simulink model "FluxModel2015a_verify_Q_zhixin"  (Simulink 10.2)
settings: Solver=VariableStepAuto  StartTime=0.0  StopTime=0.5  FixedStep=Ts  RelTol=1e-3  EnableMultiTasking=on
values from neighbouring files (symbols this model uses):
  AngleEncoderEn = 1    [parameters.m]
  AngleSpdClosedLoopEnable = 1.0    (= 1)    [parameters.m]
  CurrentLoopMax = Q14*1.2    (= 19660.8)    [parameters.m]
  EstOutMax_Speed_Q = Q14*1.2    (= 19660.8)    [parameters.m]
  Factor1 = Q15*Ts*Rb/Lq_realvalue    [parameters.m]
  Factor2 = Q15*Ts*Rs_realvalue/Lq_realvalue    (= 655.34)    [parameters.m]
  fieldweaken_Max = 0    [parameters.m]
  fieldweaken_Min = -0.5*Q14    (= -8192)    [parameters.m]
  FieldWeakenEn = 0    [parameters.m]
  fluxF = Lam_realvalue/fluxB    [parameters.m]
  fluxF_Q14 = Q14*fluxF    [parameters.m]
  Friction = 0.003    [parameters.m]
  Ib = 1.5*In    (= 24)    [parameters.m]
  Id_Ki = Q14*CurrentLoopBandwidth*Rs    [parameters.m]
  Id_Kp = Q14*CurrentLoopBandwidth*Ld    [parameters.m]
  IFCurrDecreased = 1    [parameters.m]
  IFCurrStart = 8    [parameters.m]
  IFCurrTarget = 5    [parameters.m]
  IFIdref = 0    [parameters.m]
  initial_degree = 0/Np    (= 0)    [parameters.m]
  Iq_Ki = Q14*CurrentLoopBandwidth*Rs    [parameters.m]
  Iq_Kp = Q14*CurrentLoopBandwidth*Lq    [parameters.m]
  J_realvalue = 0.69e-4    (= 6.9e-05)    [parameters.m]
  KI_fieldweaken = 0.1*Q14    (= 1638.4)    [parameters.m]
  KP_fieldweaken = 1.0*Q14    (= 16384)    [parameters.m]
  LamObserverGain = 0.8    [parameters.m]
  Ld = Ld_realvalue/Lb    [parameters.m]
  Ld_Q14 = Q14*Ld_realvalue/Lb    [parameters.m]
  Ld_realvalue = 0.25e-3    (= 0.00025)    [parameters.m]
  LowPassFilterBandWidth_Emf_Q = Q14*LowPassFilterBandWidth_Emf    (= 4915.2)    [parameters.m]
  LowPassFilterCoeff_Emf = Q15*(1/(1+LowPassFilterBandWidth_Emf*TsPu))    (= 30944.2341498)    [parameters.m]
  LowPassFilterCoeff_Spd = Q15*(1/(1+LowPassFilterBandWidth_Speed*SpeedloopTs/tb))    (= 20123.2126159)    [parameters.m]
  Lq = Lq_realvalue/Lb    [parameters.m]
  Lq_realvalue = 0.25e-3    (= 0.00025)    [parameters.m]
  MOVE = 1    [parameters.m]
  Np = 5    [parameters.m]
  Omega0 = 0.4    [parameters.m]
  OverModulationEn = 0    [parameters.m]
  PLL_KI = Q14*PLL_Bandwidth*PLL_Bandwidth    (= 655.36)    [parameters.m]
  PLL_KP = Q14*2*PLL_Bandwidth    (= 6553.6)    [parameters.m]
  PLL_OUTMax_W = Q14*1.2    (= 19660.8)    [parameters.m]
  PWM_Period = 1000    [parameters.m]
  Q14 = 16384    [parameters.m]
  Q15 = 32767    [parameters.m]
  Rs = Rs_realvalue/Rb    [parameters.m]
  Rs_Q14 = Q14*Rs_realvalue/Rb    [parameters.m]
  Rs_realvalue = 0.08    [parameters.m]
  SMO_Gain = Q14*0.85    (= 13926.4)    [parameters.m]
  SMO_I_ERROR = Q14*0.05    (= 819.2)    [parameters.m]
  SpdClosedLoopEnable = 1.0    (= 1)    [parameters.m]
  Speed_Ki = Q14*SpeedLoopBandwidth    (= 327.68)    [parameters.m]
  Speed_Kp = Q14*SpeedLoopBandwidth*JPu/fluxF    [parameters.m]
  SpeedIncreased = 0.1*Wb    (= 314.159265359)    [parameters.m]
  SpeedloopTs = 1/SpeedloopFrequency    (= 0.001)    [parameters.m]
  SpeedOutmax = 1.2*Q14    (= 19660.8)    [parameters.m]
  SpeedSwitch = 0.6*Wb    (= 1884.95559215)    [parameters.m]
  SpeedTarget = 0.8*Wb    (= 2513.27412287)    [parameters.m]
  STOP = 0    [parameters.m]
  SVCM_GAIN_Q = Q15*SVCM_GAIN    (= 26213.6)    [parameters.m]
  tb = 1/Wb    (= 0.000318309886184)    [parameters.m]
  Tf = 0    [parameters.m]
  thres = 0.1    [parameters.m]
  Ts = 1/SwitchFrequency    (= 6.25e-05)    [parameters.m]
  TsPu = Ts/tb    (= 0.196349540849)    [parameters.m]
  Vb = Un/sqrt(3)    [parameters.m]
  Vdc = 36    [parameters.m]
  Wb = 2*pi*fn    (= 3141.59265359)    [parameters.m]
undefined symbols (not defined in the file or in its folder): DiscIntNeverNeededParam, EstOutMax_Theta, magnitude, sigbuilder_block
libraries: simulink x7, powerlib x5
(part 2 of 2)

== system FluxModel2015a_verify_Q_zhixin/CurrLoop&Observer/Subsystem ==
  err : Inport
  Constant14 : Constant  Value=2*pi
  Constant15 : Constant  Value=pi
  Divide4 : Product  Inputs=*/
  Gain5 : Gain  Gain=180
  Math Function6 : Math  Operator=rem  SaturateOnIntegerOverflow=on
  err_Theta : Outport
  err_Rad : Outport  Port=2
  wires:
  Divide4/1 -> Gain5/1
  Constant15/1 -> Divide4/2
  err/1 -> Math Function6/1
  Math Function6/1 -> err_Rad/1, Divide4/1  "err_Theta"
  Constant14/1 -> Math Function6/2
  Gain5/1 -> err_Theta/1

== system FluxModel2015a_verify_Q_zhixin/CurrLoop&Observer/Subsystem1 ==
  err : Inport
  Constant14 : Constant  Value=2*pi
  Constant15 : Constant  Value=pi
  Divide4 : Product  Inputs=*/
  Gain5 : Gain  Gain=180
  Math Function6 : Math  Operator=rem  SaturateOnIntegerOverflow=on
  err_Theta : Outport
  err_Rad : Outport  Port=2
  wires:
  Gain5/1 -> err_Theta/1
  Constant14/1 -> Math Function6/2
  Math Function6/1 -> Divide4/1, err_Rad/1  "err_Theta"
  err/1 -> Math Function6/1
  Constant15/1 -> Divide4/2
  Divide4/1 -> Gain5/1

== system FluxModel2015a_verify_Q_zhixin/CurrLoop&Observer/Subsystem2 ==
  err : Inport
  Constant14 : Constant  Value=2*pi
  Constant15 : Constant  Value=pi
  Divide4 : Product  Inputs=*/
  Gain5 : Gain  Gain=180
  Math Function6 : Math  Operator=rem  SaturateOnIntegerOverflow=on
  err_Theta : Outport
  err_Rad : Outport  Port=2
  wires:
  Divide4/1 -> Gain5/1
  Constant15/1 -> Divide4/2
  err/1 -> Math Function6/1
  Math Function6/1 -> err_Rad/1, Divide4/1  "err_Theta"
  Constant14/1 -> Math Function6/2
  Gain5/1 -> err_Theta/1

== system FluxModel2015a_verify_Q_zhixin/CurrLoop&Observer/Subsystem3 ==
  err : Inport
  Constant14 : Constant  Value=2*pi
  Constant15 : Constant  Value=pi
  Divide4 : Product  Inputs=*/
  Gain5 : Gain  Gain=180
  Math Function6 : Math  Operator=rem  SaturateOnIntegerOverflow=on
  err_Theta : Outport
  err_Rad : Outport  Port=2
  wires:
  Gain5/1 -> err_Theta/1
  Constant14/1 -> Math Function6/2
  Math Function6/1 -> Divide4/1, err_Rad/1  "err_Theta"
  err/1 -> Math Function6/1
  Constant15/1 -> Divide4/2
  Divide4/1 -> Gain5/1

== system FluxModel2015a_verify_Q_zhixin/CurrLoop&Observer/Subsystem4 ==
  err : Inport
  Constant14 : Constant  Value=2*pi
  Constant15 : Constant  Value=pi
  Divide4 : Product  Inputs=*/
  Gain5 : Gain  Gain=180
  Math Function6 : Math  Operator=rem  SaturateOnIntegerOverflow=on
  err_Theta : Outport
  err_Rad : Outport  Port=2
  wires:
  Divide4/1 -> Gain5/1
  Constant15/1 -> Divide4/2
  err/1 -> Math Function6/1
  Math Function6/1 -> err_Rad/1, Divide4/1  "err_Theta"
  Constant14/1 -> Math Function6/2
  Gain5/1 -> err_Theta/1

== system FluxModel2015a_verify_Q_zhixin/CurrLoop&Observer/Subsystem5 ==
  err : Inport
  Gain1 : Gain  Gain=Wb
  Gain2 : Gain  Gain=1/(2*pi)
  Gain3 : Gain  Gain=60/Np
  err_Spd : Outport
  err_Fhz : Outport  Port=2
  wires:
  err/1 -> Gain1/1
  Gain2/1 -> err_Fhz/1, Gain3/1
  Gain1/1 -> Gain2/1
  Gain3/1 -> err_Spd/1

== system FluxModel2015a_verify_Q_zhixin/CurrLoop&Observer/Subsystem6 ==
  err : Inport
  Gain1 : Gain  Gain=Wb
  Gain2 : Gain  Gain=1/(2*pi)
  Gain3 : Gain  Gain=60/Np
  err_Spd : Outport
  err_Fhz : Outport  Port=2
  wires:
  Gain3/1 -> err_Spd/1
  Gain1/1 -> Gain2/1
  Gain2/1 -> Gain3/1, err_Fhz/1
  err/1 -> Gain1/1

== system FluxModel2015a_verify_Q_zhixin/CurrLoop&Observer/Subsystem7 ==
  err : Inport
  Gain1 : Gain  Gain=Wb
  Gain2 : Gain  Gain=1/(2*pi)
  Gain3 : Gain  Gain=60/Np
  err_Spd : Outport
  err_Fhz : Outport  Port=2
  wires:
  err/1 -> Gain1/1
  Gain2/1 -> err_Fhz/1, Gain3/1
  Gain1/1 -> Gain2/1
  Gain3/1 -> err_Spd/1

== system FluxModel2015a_verify_Q_zhixin/CurrLoop&Observer/Subsystem8 ==
  err : Inport
  Gain1 : Gain  Gain=Wb
  Gain2 : Gain  Gain=1/(2*pi)
  Gain3 : Gain  Gain=60/Np
  err_Spd : Outport
  err_Fhz : Outport  Port=2
  wires:
  Gain3/1 -> err_Spd/1
  Gain1/1 -> Gain2/1
  Gain2/1 -> Gain3/1, err_Fhz/1
  err/1 -> Gain1/1

== system FluxModel2015a_verify_Q_zhixin/CurrLoop&Observer/Subsystem9 ==
  err : Inport
  Gain1 : Gain  Gain=Wb
  Gain2 : Gain  Gain=1/(2*pi)
  Gain3 : Gain  Gain=60/Np
  err_Spd : Outport
  err_Fhz : Outport  Port=2
  wires:
  err/1 -> Gain1/1
  Gain2/1 -> err_Fhz/1, Gain3/1
  Gain1/1 -> Gain2/1
  Gain3/1 -> err_Spd/1

== system FluxModel2015a_verify_Q_zhixin/CurrLoop&Observer/ai-park ==
  UD : Inport
  UQ : Inport  Port=2
  The : Inport  Port=3
  Add : Sum
  Cos : Trigonometry  Operator=cos
  Product{,1..3} (4 alike) : Product
  Sin : Trigonometry
  Subtract : Sum  Inputs=+-
  Valpha : Outport
  Vbeta : Outport  Port=2
  wires:
  UQ/1 -> Product3/1, Product1/1
  Product1/1 -> Subtract/2
  Product3/1 -> Add/2
  Cos/1 -> Product3/2, Product/2
  Sin/1 -> Product2/2, Product1/2
  Product/1 -> Subtract/1
  Product2/1 -> Add/1
  The/1 -> Sin/1, Cos/1
  UD/1 -> Product/1, Product2/1
  Subtract/1 -> Valpha/1
  Add/1 -> Vbeta/1

== system FluxModel2015a_verify_Q_zhixin/CurrLoop&Observer/clark ==
  Iabc : Inport
  Add : Sum  Inputs=+-
  Demux2 : Demux  Outputs=3
  Gain : Gain  Gain=18919
  Gain1 : Gain  Gain=1/Q15
  Ialpha : Outport
  Ibeta : Outport  Port=2
  wires:
  Demux2/3 -> Add/2
  Add/1 -> Gain/1
  Demux2/1 -> Ialpha/1
  Demux2/2 -> Add/1
  Gain/1 -> Gain1/1
  Iabc/1 -> Demux2/1
  Gain1/1 -> Ibeta/1

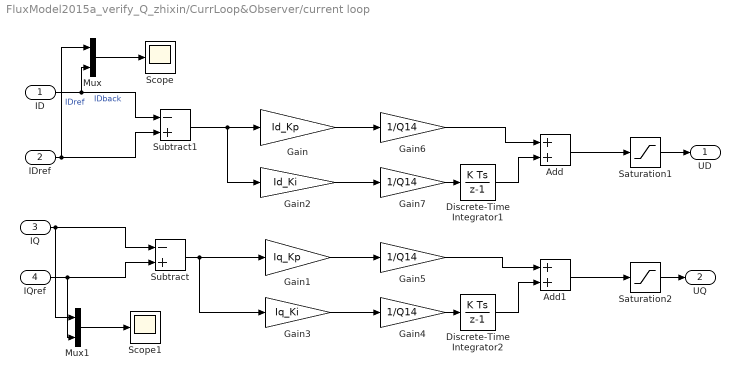
== system FluxModel2015a_verify_Q_zhixin/CurrLoop&Observer/current loop ==
  ID : Inport
  IDref : Inport  Port=2
  IQ : Inport  Port=3
  IQref : Inport  Port=4
  Add : Sum
  Add1 : Sum
  Discrete-Time Integrator1 : DiscreteIntegrator  gainval=1/tb  SampleTime=-1  LimitOutput=on  UpperSaturationLimit=CurrentLoopMax  LowerSaturationLimit=-CurrentLoopMax  ICPrevOutput=DiscIntNeverNeededParam  ICPrevScaledInput=DiscIntNeverNeededParam
  Discrete-Time Integrator2 : DiscreteIntegrator  gainval=1/tb  SampleTime=-1  LimitOutput=on  UpperSaturationLimit=CurrentLoopMax  LowerSaturationLimit=-CurrentLoopMax  ICPrevOutput=DiscIntNeverNeededParam  ICPrevScaledInput=DiscIntNeverNeededParam
  Gain : Gain  Gain=Id_Kp
  Gain1 : Gain  Gain=Iq_Kp
  Gain2 : Gain  Gain=Id_Ki
  Gain3 : Gain  Gain=Iq_Ki
  Gain{4..7} (4 alike) : Gain  Gain=1/Q14
  Mux : Mux  Inputs=2
  Mux1 : Mux  Inputs=2
  Saturation1 : Saturate  UpperLimit=CurrentLoopMax  LowerLimit=-CurrentLoopMax
  Saturation2 : Saturate  UpperLimit=CurrentLoopMax  LowerLimit=-CurrentLoopMax
  Scope : Scope
  Scope1 : Scope
  Subtract : Sum  Inputs=-+
  Subtract1 : Sum  Inputs=-+
  UD : Outport
  UQ : Outport  Port=2
  wires:
  Saturation1/1 -> UD/1
  Subtract1/1 -> Gain/1, Gain2/1
  Saturation2/1 -> UQ/1
  Gain1/1 -> Gain5/1
  Subtract/1 -> Gain3/1, Gain1/1
  Gain/1 -> Gain6/1
  Discrete-Time Integrator1/1 -> Add/2
  Discrete-Time Integrator2/1 -> Add1/2
  IQref/1 -> Mux1/2, Subtract/2
  IDref/1 -> Mux/1, Subtract1/2  "IDref"
  ID/1 -> Mux/2, Subtract1/1  "IDback"
  Mux/1 -> Scope/1
  IQ/1 -> Mux1/1, Subtract/1
  Mux1/1 -> Scope1/1
  Gain3/1 -> Gain4/1
  Add/1 -> Saturation1/1
  Add1/1 -> Saturation2/1
  Gain5/1 -> Add1/1
  Gain4/1 -> Discrete-Time Integrator2/1
  Gain6/1 -> Add/1
  Gain2/1 -> Gain7/1
  Gain7/1 -> Discrete-Time Integrator1/1

== system FluxModel2015a_verify_Q_zhixin/SVPWM/Subsystem ==
  Valpha : Inport
  Vbeta : Inport  Port=2
  Add : Sum  Inputs=+-
  Add1 : Sum  Inputs=+-
  Add2 : Sum  Inputs=+++
  Constant : Constant
  Constant1 : Constant  Value=0
  Gain : Gain  Gain=sqrt(3)
  Gain1 : Gain  Gain=-1
  Gain2 : Gain  Gain=2
  Gain3 : Gain  Gain=4
  Saturation : Saturate  UpperLimit=6  LowerLimit=1
  Switch : Switch  Criteria=u2 > Threshold
  Switch1 : Switch  Criteria=u2 > Threshold
  Switch2 : Switch  Criteria=u2 > Threshold
  N : Outport
  wires:
  Add2/1 -> Saturation/1
  Valpha/1 -> Gain/1
  Gain3/1 -> Add2/2
  Gain2/1 -> Add2/1
  Add1/1 -> Switch1/2
  Gain1/1 -> Add1/1
  Switch1/1 -> Gain3/1
  Switch2/1 -> Add2/3
  Switch/1 -> Gain2/1
  Add/1 -> Switch/2
  Gain/1 -> Gain1/1, Add/1
  Constant1/1 -> Switch/3, Switch1/3, Switch2/3
  Constant/1 -> Switch1/1, Switch2/1, Switch/1
  Vbeta/1 -> Add1/2, Add/2, Switch2/2
  Saturation/1 -> N/1

== system FluxModel2015a_verify_Q_zhixin/SVPWM/Subsystem1 ==
  T : Inport
  In2 : Inport  Port=2
  In3 : Inport  Port=3
  Vdc : Inport  Port=4
  Add : Sum
  Add1 : Sum  Inputs=+-
  Divide : Product  Inputs=**/
  Divide1 : Product  Inputs=**/
  Divide2 : Product  Inputs=**/
  Gain : Gain  Gain=1.732
  Gain1 : Gain  Gain=1.5
  Gain2 : Gain  Gain=0.5
  x : Outport
  Y : Outport  Port=2
  Z : Outport  Port=3
  wires:
  Divide2/1 -> Z/1
  Divide1/1 -> Y/1
  Divide/1 -> x/1
  In3/1 -> Gain1/1
  In2/1 -> Gain/1
  Add1/1 -> Divide2/2
  Add/1 -> Divide1/2
  Gain1/1 -> Add1/2, Add/2
  Gain2/1 -> Add1/1, Add/1
  Gain/1 -> Gain2/1, Divide/2
  Vdc/1 -> Divide/3, Divide1/3, Divide2/3
  T/1 -> Divide2/1, Divide1/1, Divide/1

== system FluxModel2015a_verify_Q_zhixin/SVPWM/Subsystem2 ==
  In1 : Inport
  In2 : Inport  Port=2
  In3 : Inport  Port=3
  In4 : Inport  Port=4
  Gain : Gain  Gain=-1
  Gain1 : Gain  Gain=-1
  Gain2 : Gain  Gain=-1
  Multiport Switch : MultiPortSwitch  Inputs=6
  Multiport Switch1 : MultiPortSwitch  Inputs=6
  T1 : Outport
  T2 : Outport  Port=2
  wires:
  Multiport Switch1/1 -> T2/1
  Multiport Switch/1 -> T1/1
  Gain2/1 -> Multiport Switch/7, Multiport Switch1/6
  Gain1/1 -> Multiport Switch1/3, Multiport Switch/5
  In2/1 -> Gain1/1, Multiport Switch/6, Multiport Switch1/4
  Gain/1 -> Multiport Switch1/7, Multiport Switch/4
  In3/1 -> Gain2/1, Multiport Switch1/2, Multiport Switch/3
  In4/1 -> Gain/1, Multiport Switch/2, Multiport Switch1/5
  In1/1 -> Multiport Switch/1, Multiport Switch1/1

== system FluxModel2015a_verify_Q_zhixin/SVPWM/Subsystem3 ==
  T1 : Inport
  T : Inport  Port=2
  T2 : Inport  Port=3
  Add : Sum  Inputs=--+
  Add1 : Sum
  Add2 : Sum
  Gain : Gain  Gain=0.25
  Gain1 : Gain  Gain=0.5
  Gain2 : Gain  Gain=0.5
  Saturation : Saturate  UpperLimit=Ts/2  LowerLimit=0
  Ta : Outport
  Tb : Outport  Port=2
  Tc : Outport  Port=3
  wires:
  T/1 -> Add/3
  Gain2/1 -> Add2/2
  Gain1/1 -> Add1/2
  Add/1 -> Gain/1
  T2/1 -> Add/2, Gain2/1
  T1/1 -> Add/1, Gain1/1
  Gain/1 -> Ta/1, Add1/1
  Add1/1 -> Tb/1, Add2/1
  Add2/1 -> Tc/1

== system FluxModel2015a_verify_Q_zhixin/SVPWM/Subsystem4 ==
  In1 : Inport
  In2 : Inport  Port=2
  In3 : Inport  Port=3
  In4 : Inport  Port=4
  Multiport Switch : MultiPortSwitch  Inputs=6
  Multiport Switch1 : MultiPortSwitch  Inputs=6
  Multiport Switch2 : MultiPortSwitch  Inputs=6
  Tcm1 : Outport
  Tcm2 : Outport  Port=2
  Tcm3 : Outport  Port=3
  wires:
  Multiport Switch2/1 -> Tcm3/1
  Multiport Switch1/1 -> Tcm2/1
  Multiport Switch/1 -> Tcm1/1
  In1/1 -> Multiport Switch2/1, Multiport Switch1/1, Multiport Switch/1
  In4/1 -> Multiport Switch1/3, Multiport Switch1/7, Multiport Switch2/2, Multiport Switch2/4, Multiport Switch/5, Multiport Switch/6
  In2/1 -> Multiport Switch/4, Multiport Switch/3, Multiport Switch1/2, Multiport Switch1/6, Multiport Switch2/5, Multiport Switch2/7
  In3/1 -> Multiport Switch/2, Multiport Switch/7, Multiport Switch1/4, Multiport Switch1/5, Multiport Switch2/3, Multiport Switch2/6

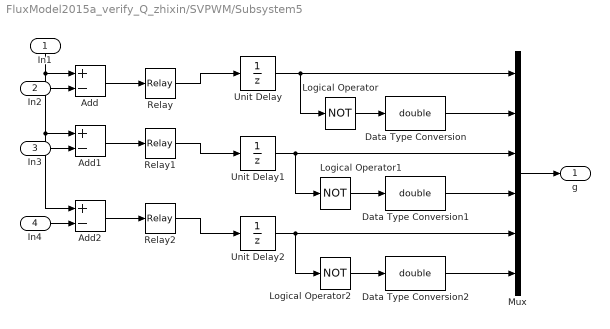
== system FluxModel2015a_verify_Q_zhixin/SVPWM/Subsystem5 ==
  In1 : Inport
  In2 : Inport  Port=2
  In3 : Inport  Port=3
  In4 : Inport  Port=4
  Add : Sum  Inputs=+-
  Add1 : Sum  Inputs=+-
  Add2 : Sum  Inputs=+-
  Data Type Conversion : DataTypeConversion  OutDataTypeStr=double
  Data Type Conversion1 : DataTypeConversion  OutDataTypeStr=double
  Data Type Conversion2 : DataTypeConversion  OutDataTypeStr=double
  Logical Operator : Logic  Operator=NOT  AllPortsSameDT=off  OutDataTypeStr=boolean
  Logical Operator1 : Logic  Operator=NOT  AllPortsSameDT=off  OutDataTypeStr=boolean
  Logical Operator2 : Logic  Operator=NOT  AllPortsSameDT=off  OutDataTypeStr=boolean
  Mux : Mux  Inputs=6
  Relay : Relay  OffSwitchValue=-eps
  Relay1 : Relay  OffSwitchValue=-eps
  Relay2 : Relay  OffSwitchValue=-eps
  Unit Delay : UnitDelay  SampleTime=-1  HasFrameUpgradeWarning=on
  Unit Delay1 : UnitDelay  SampleTime=-1  HasFrameUpgradeWarning=on
  Unit Delay2 : UnitDelay  SampleTime=-1  HasFrameUpgradeWarning=on
  g : Outport
  wires:
  In4/1 -> Add2/2
  In3/1 -> Add1/2
  In2/1 -> Add/2
  Relay2/1 -> Unit Delay2/1
  Relay1/1 -> Unit Delay1/1
  Relay/1 -> Unit Delay/1
  Unit Delay2/1 -> Mux/5, Logical Operator2/1
  Unit Delay1/1 -> Mux/3, Logical Operator1/1
  Unit Delay/1 -> Logical Operator/1, Mux/1
  Mux/1 -> g/1
  Data Type Conversion/1 -> Mux/2
  Data Type Conversion1/1 -> Mux/4
  Data Type Conversion2/1 -> Mux/6
  Logical Operator2/1 -> Data Type Conversion2/1
  Logical Operator1/1 -> Data Type Conversion1/1
  Logical Operator/1 -> Data Type Conversion/1
  Add1/1 -> Relay1/1
  Add2/1 -> Relay2/1
  Add/1 -> Relay/1
  In1/1 -> Add/1, Add2/1, Add1/1

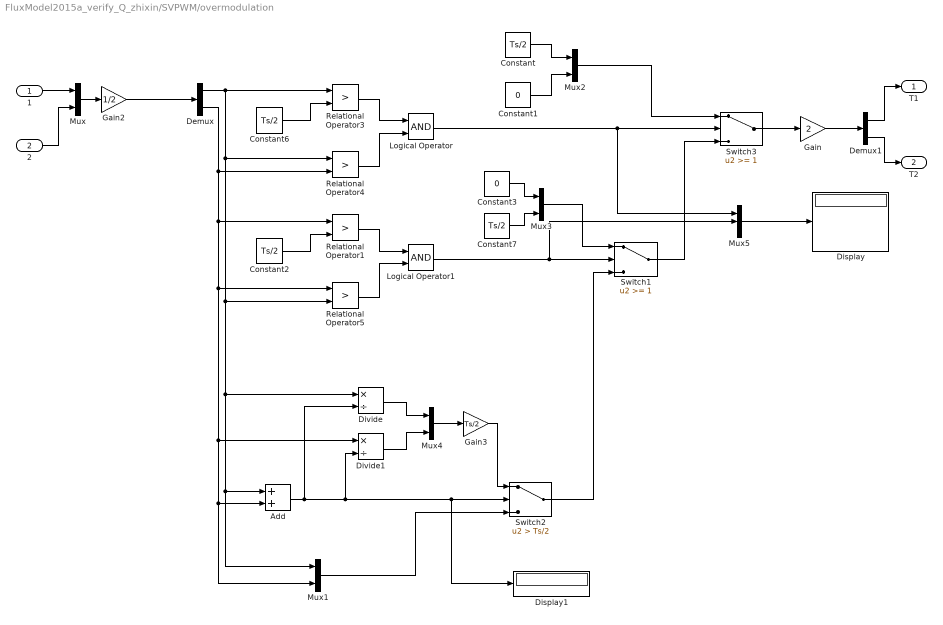
== system FluxModel2015a_verify_Q_zhixin/SVPWM/overmodulation ==
  1 : Inport
  2 : Inport  Port=2
  Add : Sum
  Constant{,2,6..7} (4 alike) : Constant  Value=Ts/2
  Constant1 : Constant  Value=0
  Constant3 : Constant  Value=0
  Demux : Demux  Outputs=2
  Demux1 : Demux  Outputs=2
  Display : Display
  Display1 : Display
  Divide : Product  Inputs=*/
  Divide1 : Product  Inputs=*/
  Gain : Gain  Gain=2
  Gain2 : Gain  Gain=1/2
  Gain3 : Gain  Gain=Ts/2
  Logical Operator : Logic  AllPortsSameDT=off  OutDataTypeStr=boolean
  Logical Operator1 : Logic  AllPortsSameDT=off  OutDataTypeStr=boolean
  Mux{,1..4} (5 alike) : Mux  Inputs=2
  Mux5 : Mux  Inputs=3
  Relational Operator{1,3..5} (4 alike) : RelationalOperator  Operator=>  OutDataTypeStr=boolean  RndMeth=Simplest
  Switch1 : Switch  Threshold=1
  Switch2 : Switch  Criteria=u2 > Threshold  Threshold=Ts/2
  Switch3 : Switch  Threshold=1
  T1 : Outport
  T2 : Outport  Port=2
  wires:
  Switch1/1 -> Switch3/3
  Constant3/1 -> Mux3/1
  Divide/1 -> Mux4/1
  Mux/1 -> Gain2/1
  Logical Operator1/1 -> Mux5/2, Switch1/2
  Switch2/1 -> Switch1/3
  Add/1 -> Divide/2, Divide1/2, Display1/1, Switch2/2
  Demux/1 -> Relational Operator4/1, Relational Operator5/2, Divide/1, Mux1/1, Add/1, Relational Operator3/1
  Mux4/1 -> Gain3/1
  Divide1/1 -> Mux4/2
  Relational Operator1/1 -> Logical Operator1/1
  Constant7/1 -> Mux3/2
  Demux/2 -> Relational Operator4/2, Relational Operator1/1, Relational Operator5/1, Divide1/1, Mux1/2, Add/2
  Mux2/1 -> Switch3/1
  Gain2/1 -> Demux/1
  Relational Operator3/1 -> Logical Operator/1
  Relational Operator4/1 -> Logical Operator/2
  Relational Operator5/1 -> Logical Operator1/2
  Constant/1 -> Mux2/1
  Constant6/1 -> Relational Operator3/2
  Gain3/1 -> Switch2/1
  Constant2/1 -> Relational Operator1/2
  Constant1/1 -> Mux2/2
  Logical Operator/1 -> Mux5/1, Switch3/2
  Mux3/1 -> Switch1/1
  1/1 -> Mux/1
  2/1 -> Mux/2
  Demux1/1 -> T1/1
  Demux1/2 -> T2/1
  Mux1/1 -> Switch2/3
  Mux5/1 -> Display/1
  Switch3/1 -> Gain/1
  Gain/1 -> Demux1/1

== system FluxModel2015a_verify_Q_zhixin/SpeedLoop/FieldWeaken ==
  UDUQ : Inport
  Add4 : Sum
  Add5 : Sum  Inputs=+-
  Add6 : Sum
  Constant : Constant  Value=Vdc/sqrt(3)/Vb
  Delay : Delay  DelayLength=1
  Demux : Demux  Outputs=2
  Discrete-Time Integrator : DiscreteIntegrator  gainval=1.0/tb  SampleTime=-1  LimitOutput=on  UpperSaturationLimit=fieldweaken_Max  LowerSaturationLimit=fieldweaken_Min  ICPrevOutput=DiscIntNeverNeededParam  ICPrevScaledInput=DiscIntNeverNeededParam
  Gain : Gain  Gain=KP_fieldweaken
  Gain1 : Gain  Gain=Q14
  Gain2 : Gain  Gain=KI_fieldweaken
  Gain3 : Gain  Gain=1/Q14
  Gain4 : Gain  Gain=1/Q14
  Saturation : Saturate  UpperLimit=fieldweaken_Max  LowerLimit=fieldweaken_Min
  Sqrt1 : Sqrt  SaturateOnIntegerOverflow=on
  Square1 : Math  Operator=square  SaturateOnIntegerOverflow=on
  ID : Outport
  wires:
  Delay/1 -> Square1/1
  Demux/2 -> Add4/2
  Add5/1 -> Gain2/1, Gain/1
  Add4/1 -> Sqrt1/1
  Square1/1 -> Demux/1
  Add6/1 -> Saturation/1
  Discrete-Time Integrator/1 -> Add6/2
  UDUQ/1 -> Delay/1
  Saturation/1 -> ID/1
  Demux/1 -> Add4/1
  Constant/1 -> Gain1/1
  Gain/1 -> Gain3/1
  Gain2/1 -> Gain4/1
  Sqrt1/1 -> Add5/2
  Gain3/1 -> Add6/1
  Gain4/1 -> Discrete-Time Integrator/1
  Gain1/1 -> Add5/1

== system FluxModel2015a_verify_Q_zhixin/SpeedLoop/LowPassFilter2 ==
  Input : Inport
  Add : Sum  Inputs=+-
  Add1 : Sum
  Constant : Constant  Value=32767
  Constant1 : Constant  Value=LowPassFilterCoeff_Spd
  Delay6 : Delay  DelayLength=1
  Gain : Gain  Gain=LowPassFilterCoeff_Spd
  Gain3 : Gain  Gain=1/Q15
  Product : Product
  Output : Outport
  wires:
  Add1/1 -> Gain3/1
  Gain/1 -> Add1/2
  Product/1 -> Add1/1
  Add/1 -> Product/2
  Input/1 -> Product/1
  Constant1/1 -> Add/2
  Constant/1 -> Add/1
  Delay6/1 -> Gain/1
  Gain3/1 -> Output/1, Delay6/1

== system FluxModel2015a_verify_Q_zhixin/SpeedLoop/MTPA ==
  IQ : Inport
  Add4 : Sum
  Add5 : Sum  Inputs=+-
  Constant : Constant  Value=(fluxF^2)/(4*((Ld-Lq)^2))
  Constant1 : Constant  Value=fluxF/(2*(Lq-Ld))
  Sqrt1 : Sqrt  SaturateOnIntegerOverflow=on
  Square : Math  Operator=square  SaturateOnIntegerOverflow=on
  ID : Outport
  wires:
  Sqrt1/1 -> Add5/2
  Square/1 -> Add4/1
  Add4/1 -> Sqrt1/1
  Constant1/1 -> Add5/1
  Constant/1 -> Add4/2
  Add5/1 -> ID/1
  IQ/1 -> Square/1

== system FluxModel2015a_verify_Q_zhixin/SpeedLoop/OpenLoop ==
  CurrDecreased : Inport
  CurrTarget : Inport  Port=2
  CurrStart : Inport  Port=3
  SpdIncreased : Inport  Port=4
  SpdTarget : Inport  Port=5
  Add : Sum
  Add1 : Sum  Inputs=+-
  Constant : Constant  Value=IFIdref
  Delay : Delay  DelayLength=1
  Delay1 : Delay  DelayLength=1  InitialCondition=IFCurrStart
  Gain : Gain  Gain=1/Wb
  Gain1 : Gain  Gain=1/Ib
  Gain8 : Gain  Gain=1/Ib
  Scope : Scope
  Switch2 : Switch  Criteria=u2 > Threshold  Threshold=SpeedSwitch
  Switch3 : Switch  Criteria=u2 > Threshold  Threshold=IFCurrTarget
  Switch4 : Switch  Criteria=u2 > Threshold  Threshold=SpeedTarget
  OpenLoopIQ : Outport
  OpenLoopID : Outport  Port=2
  OpenLoopSpeed : Outport  Port=3
  wires:
  Delay1/1 -> Switch3/1, Switch3/2, Add1/1
  CurrDecreased/1 -> Add1/2
  Add1/1 -> Delay1/1
  SpdTarget/1 -> Switch4/1
  Delay/1 -> Switch4/3, Switch4/2, Switch2/2, Add/1
  Switch3/1 -> Scope/1, Switch2/1
  SpdIncreased/1 -> Add/2
  Add/1 -> Delay/1
  CurrStart/1 -> Switch2/3
  Switch2/1 -> Gain8/1
  Gain8/1 -> OpenLoopIQ/1
  Switch4/1 -> Gain/1
  Gain/1 -> OpenLoopSpeed/1
  CurrTarget/1 -> Switch3/3
  Constant/1 -> Gain1/1
  Gain1/1 -> OpenLoopID/1

== system FluxModel2015a_verify_Q_zhixin/CurrLoop&Observer/Nonlinear Observer/PLL ==
  error : Inport
  Add14 : Sum
  Discrete-Time Integrator2 : DiscreteIntegrator  gainval=1.0/tb  SampleTime=-1  LimitOutput=on  UpperSaturationLimit=PLL_OUTMax_W  LowerSaturationLimit=-PLL_OUTMax_W  ICPrevOutput=DiscIntNeverNeededParam  ICPrevScaledInput=DiscIntNeverNeededParam
  Discrete-Time Integrator4 : DiscreteIntegrator  gainval=1.0/tb  SampleTime=-1  UpperSaturationLimit=PLL_OUTMax_W  LowerSaturationLimit=-PLL_OUTMax_W  ICPrevOutput=DiscIntNeverNeededParam  ICPrevScaledInput=DiscIntNeverNeededParam
  Gain : Gain  Gain=PLL_KP
  Gain7 : Gain  Gain=PLL_KI
  Saturation : Saturate  UpperLimit=PLL_OUTMax_W  LowerLimit=-PLL_OUTMax_W
  est_theta : Outport
  est_speed : Outport  Port=2
  wires:
  Gain7/1 -> Discrete-Time Integrator2/1
  Discrete-Time Integrator4/1 -> est_theta/1
  Add14/1 -> Saturation/1
  Saturation/1 -> est_speed/1, Discrete-Time Integrator4/1
  error/1 -> Gain7/1, Gain/1
  Discrete-Time Integrator2/1 -> Add14/2
  Gain/1 -> Add14/1

== system FluxModel2015a_verify_Q_zhixin/CurrLoop&Observer/Nonlinear Observer/clark ==
  Iabc : Inport
  Add : Sum  Inputs=+-
  Demux2 : Demux  Outputs=3
  Gain : Gain  Gain=1/sqrt(3)
  Ialpha : Outport
  Ibeta : Outport  Port=2
  wires:
  Demux2/3 -> Add/2
  Add/1 -> Gain/1
  Demux2/1 -> Ialpha/1
  Demux2/2 -> Add/1
  Gain/1 -> Ibeta/1
  Iabc/1 -> Demux2/1

== system FluxModel2015a_verify_Q_zhixin/CurrLoop&Observer/PLLObserver/PLL ==
  error : Inport
  Add14 : Sum
  Discrete-Time Integrator2 : DiscreteIntegrator  gainval=1.0/tb  SampleTime=-1  LimitOutput=on  UpperSaturationLimit=PLL_OUTMax_W  LowerSaturationLimit=-PLL_OUTMax_W  ICPrevOutput=DiscIntNeverNeededParam  ICPrevScaledInput=DiscIntNeverNeededParam
  Discrete-Time Integrator4 : DiscreteIntegrator  gainval=1.0/tb  SampleTime=-1  UpperSaturationLimit=PLL_OUTMax_W  LowerSaturationLimit=-PLL_OUTMax_W  ICPrevOutput=DiscIntNeverNeededParam  ICPrevScaledInput=DiscIntNeverNeededParam
  Gain : Gain  Gain=PLL_KP
  Gain7 : Gain  Gain=PLL_KI
  Saturation1 : Saturate  UpperLimit=PLL_OUTMax_W  LowerLimit=-PLL_OUTMax_W
  est_theta : Outport
  est_speed : Outport  Port=2
  wires:
  Gain7/1 -> Discrete-Time Integrator2/1
  Discrete-Time Integrator4/1 -> est_theta/1
  Add14/1 -> Saturation1/1
  Saturation1/1 -> est_speed/1, Discrete-Time Integrator4/1
  error/1 -> Gain7/1, Gain/1
  Discrete-Time Integrator2/1 -> Add14/2
  Gain/1 -> Add14/1

== system FluxModel2015a_verify_Q_zhixin/CurrLoop&Observer/PLLObserver/PLL1 ==
  error : Inport
  Add14 : Sum
  Discrete-Time Integrator2 : DiscreteIntegrator  gainval=1.0/tb  SampleTime=-1  LimitOutput=on  UpperSaturationLimit=PLL_OUTMax_W  LowerSaturationLimit=-PLL_OUTMax_W  ICPrevOutput=DiscIntNeverNeededParam  ICPrevScaledInput=DiscIntNeverNeededParam
  Discrete-Time Integrator4 : DiscreteIntegrator  gainval=1.0/tb  SampleTime=-1  UpperSaturationLimit=PLL_OUTMax_W  LowerSaturationLimit=-PLL_OUTMax_W  ICPrevOutput=DiscIntNeverNeededParam  ICPrevScaledInput=DiscIntNeverNeededParam
  Gain : Gain  Gain=PLL_KP
  Gain7 : Gain  Gain=PLL_KI
  Saturation1 : Saturate  UpperLimit=PLL_OUTMax_W  LowerLimit=-PLL_OUTMax_W
  est_theta : Outport
  est_speed : Outport  Port=2
  wires:
  Gain7/1 -> Discrete-Time Integrator2/1
  Discrete-Time Integrator4/1 -> est_theta/1
  Add14/1 -> Saturation1/1
  Saturation1/1 -> est_speed/1, Discrete-Time Integrator4/1
  error/1 -> Gain7/1, Gain/1
  Discrete-Time Integrator2/1 -> Add14/2
  Gain/1 -> Add14/1

== system FluxModel2015a_verify_Q_zhixin/CurrLoop&Observer/ReactivePowerObserver/PLL1 ==
  error : Inport
  Add14 : Sum
  Discrete-Time Integrator2 : DiscreteIntegrator  gainval=1.0/tb  SampleTime=-1  LimitOutput=on  UpperSaturationLimit=PLL_OUTMax_W  LowerSaturationLimit=-PLL_OUTMax_W  ICPrevOutput=DiscIntNeverNeededParam  ICPrevScaledInput=DiscIntNeverNeededParam
  Discrete-Time Integrator4 : DiscreteIntegrator  gainval=1.0/tb  SampleTime=-1  UpperSaturationLimit=PLL_OUTMax_W  LowerSaturationLimit=-PLL_OUTMax_W  ICPrevOutput=DiscIntNeverNeededParam  ICPrevScaledInput=DiscIntNeverNeededParam
  Gain : Gain  Gain=PLL_KP
  Gain7 : Gain  Gain=PLL_KI
  Saturation : Saturate  UpperLimit=PLL_OUTMax_W  LowerLimit=-PLL_OUTMax_W
  est_theta : Outport
  est_speed : Outport  Port=2
  wires:
  Gain7/1 -> Discrete-Time Integrator2/1
  Discrete-Time Integrator4/1 -> est_theta/1
  Add14/1 -> Saturation/1
  Saturation/1 -> est_speed/1, Discrete-Time Integrator4/1
  error/1 -> Gain7/1, Gain/1
  Discrete-Time Integrator2/1 -> Add14/2
  Gain/1 -> Add14/1

== system FluxModel2015a_verify_Q_zhixin/CurrLoop&Observer/SMO/LowPassFilter1 ==
  Input : Inport
  Add : Sum  Inputs=+-
  Add1 : Sum
  Constant : Constant  Value=32767
  Constant1 : Constant  Value=LowPassFilterCoeff_Emf
  Delay6 : Delay  DelayLength=1
  Gain : Gain  Gain=LowPassFilterCoeff_Emf
  Gain3 : Gain  Gain=1/Q15
  Product : Product
  Output : Outport
  wires:
  Delay6/1 -> Gain/1
  Constant/1 -> Add/1
  Constant1/1 -> Add/2
  Input/1 -> Product/1
  Add/1 -> Product/2
  Product/1 -> Add1/1
  Gain/1 -> Add1/2
  Add1/1 -> Gain3/1
  Gain3/1 -> Delay6/1, Output/1

== system FluxModel2015a_verify_Q_zhixin/CurrLoop&Observer/SMO/LowPassFilter2 ==
  Input : Inport
  Add : Sum  Inputs=+-
  Add1 : Sum
  Constant : Constant  Value=32767
  Constant1 : Constant  Value=LowPassFilterCoeff_Emf
  Delay6 : Delay  DelayLength=1
  Gain : Gain  Gain=LowPassFilterCoeff_Emf
  Gain3 : Gain  Gain=1/Q15
  Product : Product
  Output : Outport
  wires:
  Add1/1 -> Gain3/1
  Gain/1 -> Add1/2
  Product/1 -> Add1/1
  Add/1 -> Product/2
  Input/1 -> Product/1
  Constant1/1 -> Add/2
  Constant/1 -> Add/1
  Delay6/1 -> Gain/1
  Gain3/1 -> Output/1, Delay6/1

== system FluxModel2015a_verify_Q_zhixin/CurrLoop&Observer/SMO/PLL2 ==
  error : Inport
  Add14 : Sum
  Discrete-Time Integrator2 : DiscreteIntegrator  gainval=1.0/tb  SampleTime=-1  LimitOutput=on  UpperSaturationLimit=PLL_OUTMax_W  LowerSaturationLimit=-PLL_OUTMax_W  ICPrevOutput=DiscIntNeverNeededParam  ICPrevScaledInput=DiscIntNeverNeededParam
  Discrete-Time Integrator4 : DiscreteIntegrator  gainval=1.0/tb  SampleTime=-1  UpperSaturationLimit=1024  LowerSaturationLimit=0  ICPrevOutput=DiscIntNeverNeededParam  ICPrevScaledInput=DiscIntNeverNeededParam
  Gain : Gain  Gain=PLL_KP
  Gain1 : Gain  Gain=1/Q14
  Gain2 : Gain  Gain=1/Q14
  Gain3 : Gain  Gain=10430/Q14
  Gain7 : Gain  Gain=PLL_KI
  Saturation : Saturate  UpperLimit=PLL_OUTMax_W  LowerLimit=-PLL_OUTMax_W
  Scope : Scope
  est_theta : Outport
  est_speed : Outport  Port=2
  wires:
  Gain/1 -> Gain1/1
  Discrete-Time Integrator2/1 -> Add14/2
  error/1 -> Gain/1, Gain7/1
  Gain7/1 -> Gain2/1
  Gain1/1 -> Add14/1
  Gain2/1 -> Discrete-Time Integrator2/1
  Add14/1 -> Saturation/1
  Discrete-Time Integrator4/1 -> Gain3/1
  Gain3/1 -> est_theta/1
  Saturation/1 -> Scope/1, Discrete-Time Integrator4/1, est_speed/1

== system FluxModel2015a_verify_Q_zhixin/CurrLoop&Observer/SMO/clark1 ==
  Iabc : Inport
  Add : Sum  Inputs=+-
  Demux2 : Demux  Outputs=3
  Gain1 : Gain  Gain=18919
  Gain2 : Gain  Gain=1/Q15
  Ialpha : Outport
  Ibeta : Outport  Port=2
  wires:
  Iabc/1 -> Demux2/1
  Gain2/1 -> Ibeta/1
  Demux2/2 -> Add/1
  Demux2/1 -> Ialpha/1
  Add/1 -> Gain1/1
  Demux2/3 -> Add/2
  Gain1/1 -> Gain2/1

== system FluxModel2015a_verify_Q_zhixin/CurrLoop&Observer/SVCM/clark1 ==
  Iabc : Inport
  Add : Sum  Inputs=+-
  Demux2 : Demux  Outputs=3
  Gain : Gain  Gain=18919
  Gain1 : Gain  Gain=1/Q15
  Ialpha : Outport
  Ibeta : Outport  Port=2
  wires:
  Demux2/3 -> Add/2
  Add/1 -> Gain/1
  Demux2/1 -> Ialpha/1
  Demux2/2 -> Add/1
  Iabc/1 -> Demux2/1
  Gain/1 -> Gain1/1
  Gain1/1 -> Ibeta/1

== system FluxModel2015a_verify_Q_zhixin/CurrLoop&Observer/SVCM/park0 ==
  alpha : Inport
  beta : Inport  Port=2
  theta : Inport  Port=3
  Add : Sum
  Add1 : Sum  Inputs=+-
  Product{,1..3} (4 alike) : Product
  Sin : Trigonometry
  Sin1 : Trigonometry  Operator=cos
  D : Outport
  Q : Outport  Port=2
  wires:
  theta/1 -> Sin1/1, Sin/1
  Product2/1 -> Add1/1
  Sin/1 -> Product3/2, Product1/2
  Add/1 -> D/1
  Product3/1 -> Add1/2
  Sin1/1 -> Product2/2, Product/2
  beta/1 -> Product1/1, Product2/1
  Product1/1 -> Add/2
  Product/1 -> Add/1
  alpha/1 -> Product3/1, Product/1
  Add1/1 -> Q/1

== system FluxModel2015a_verify_Q_zhixin/CurrLoop&Observer/SVCM/park1 ==
  alpha : Inport
  beta : Inport  Port=2
  theta : Inport  Port=3
  Add : Sum
  Add1 : Sum  Inputs=+-
  Product{,1..3} (4 alike) : Product
  Sin : Trigonometry
  Sin1 : Trigonometry  Operator=cos
  D : Outport
  Q : Outport  Port=2
  wires:
  Add1/1 -> Q/1
  alpha/1 -> Product/1, Product3/1
  Product/1 -> Add/1
  Product1/1 -> Add/2
  beta/1 -> Product2/1, Product1/1
  Sin1/1 -> Product/2, Product2/2
  Product3/1 -> Add1/2
  Add/1 -> D/1
  Sin/1 -> Product1/2, Product3/2
  Product2/1 -> Add1/1
  theta/1 -> Sin/1, Sin1/1

== stateflow chart Chart ==
  settings: executeAtInitialization=1
  state STOP
    en:
    MotionState = STOP;
    du:
    MotionState = STOP;
  state Move
    en:
    MotionState = MOVE;
    du:
    MotionState = MOVE;
  (default) -> STOP
  STOP -> Move : [Speed>=thres]
  Move -> STOP : [Speed<thres]
  data: Speed input double; MotionState output boolean; STOP parameter boolean; MOVE parameter boolean; thres parameter single

== matlab function Note/MATLAB Function ==
  data: u input double; y output double
function y = fcn(u)

y = u;
